simulink library "lib_all"  (Simulink 7.6)
settings: Solver=ode45  SolverMode=SingleTasking  StartTime=0.0  StopTime=10.0  RelTol=1e-3
values from neighbouring files (symbols this model uses):
  map_lib_03 = [6.8679 7.0159 7.4571 8.1849 9.1892 10.456 11.97 13.71 15.655 17.781 20.063 22.474 ... (803 elements, 73x11)]    [scr_simdata.mat]    (also in scr_simdata_all.mat)
  map_lib_06 = [2.5784 2.6727 2.9588 3.4453 4.1444 5.068 6.226 7.6242 9.2634 11.139 13.24 15.552 ... (803 elements, 73x11)]    [scr_simdata.mat]    (also in scr_simdata_all.mat)
  map_lib_09 = [0.0025784 0.0026727 0.0029588 0.0034453 0.0041444 0.005068 0.006226 0.0076242 0.0092634 0.011139 0.01324 0.015552 ... (803 elements, 73x11)]    [scr_simdata.mat]    (also in scr_simdata_all.mat)
  map_lib_10 = [2.74 2.75 2.76 2.77 2.78 2.79 2.8 2.81 2.82 2.83 2.84 2.85 ... (81 elements, 1x81)]    [scr_simdata.mat]    (also in scr_simdata_all.mat)
  map_lib_11 = [1.1983 1.2027 1.207 1.2113 1.2156 1.2198 1.2241 1.2283 1.2326 1.2368 1.241 1.2451 ... (81 elements, 1x81)]    [scr_simdata.mat]    (also in scr_simdata_all.mat)
  map_lib_12 = [2.74 2.75 2.76 2.77 2.78 2.79 2.8 2.81 2.82 2.83 2.84 2.85 ... (81 elements, 1x81)]    [scr_simdata.mat]    (also in scr_simdata_all.mat)
  map_lib_13 = [1.74 1.75 1.76 1.77 1.78 1.79 1.8 1.81 1.82 1.83 1.84 1.85 ... (81 elements, 1x81)]    [scr_simdata.mat]    (also in scr_simdata_all.mat)
  map_lib_16 = [0.00048828125 1.6159 1.8962 2.0821 2.2251 2.3426 2.4433 2.5318 2.611 2.683 2.749 2.8101 ... (1419 elements, 129x11)]    [scr_simdata.mat]    (also in scr_simdata_all.mat)
  map_mr2_01 = [0 800 1600 2400 3200 4000]    [scr_simdata.mat]    (also in scr_simdata_all.mat)
  map_mr2_02 = [1.32 1.32 1.33 1.34 1.36 1.38]    [scr_simdata.mat]    (also in scr_simdata_all.mat)
  map_mr2_03 = [0 800 1600 2400 3200 4000]    [scr_simdata.mat]    (also in scr_simdata_all.mat)
  map_mr2_04 = [1.32 1.32 1.33 1.34 1.36 1.38]    [scr_simdata.mat]    (also in scr_simdata_all.mat)
  map_mr2_05 = [0 800 1600 2400 3200 4000]    [scr_simdata.mat]    (also in scr_simdata_all.mat)
  map_mr2_06 = [1.32 1.32 1.33 1.34 1.36 1.38]    [scr_simdata.mat]    (also in scr_simdata_all.mat)
  Parameter1 = 6    [scr_simdata.mat]    (also in scr_simdata_all.mat)
  Parameter101 = 30    [scr_simdata.mat]    (also in scr_simdata_all.mat)
  Parameter102 = 12    [scr_simdata.mat]    (also in scr_simdata_all.mat)
  Parameter103 = -240    [scr_simdata.mat]    (also in scr_simdata_all.mat)
  Parameter108 = 48    [scr_simdata.mat]    (also in scr_simdata_all.mat)
  Parameter120 = 16    [scr_simdata.mat]    (also in scr_simdata_all.mat)
  Parameter121 = 87    [scr_simdata.mat]    (also in scr_simdata_all.mat)
  Parameter123 = 78    [scr_simdata.mat]    (also in scr_simdata_all.mat)
  Parameter124 = 60    [scr_simdata.mat]    (also in scr_simdata_all.mat)
  Parameter125 = -480    [scr_simdata.mat]    (also in scr_simdata_all.mat)
  Parameter130 = 96    [scr_simdata.mat]    (also in scr_simdata_all.mat)
  Parameter131 = 3    [scr_simdata.mat]    (also in scr_simdata_all.mat)
  Parameter144 = 3    [scr_simdata.mat]    (also in scr_simdata_all.mat)
  Parameter18 = 128    [scr_simdata.mat]    (also in scr_simdata_all.mat)
  Parameter19 = 16    [scr_simdata.mat]    (also in scr_simdata_all.mat)
  Parameter2 = 12    [scr_simdata.mat]    (also in scr_simdata_all.mat)
  Parameter20 = 7    [scr_simdata.mat]    (also in scr_simdata_all.mat)
  Parameter21 = 4    [scr_simdata.mat]    (also in scr_simdata_all.mat)
  Parameter3 = 18    [scr_simdata.mat]    (also in scr_simdata_all.mat)
  Parameter32 = 1    [scr_simdata.mat]    (also in scr_simdata_all.mat)
  Parameter34 = 24    [scr_simdata.mat]    (also in scr_simdata_all.mat)
  Parameter4 = 138    [scr_simdata.mat]    (also in scr_simdata_all.mat)
  Parameter42 = 48    [scr_simdata.mat]    (also in scr_simdata_all.mat)
  Parameter5 = 135    [scr_simdata.mat]    (also in scr_simdata_all.mat)
  Parameter55 = 0    [scr_simdata.mat]    (also in scr_simdata_all.mat)
  Parameter56 = 0    [scr_simdata.mat]    (also in scr_simdata_all.mat)
  Parameter57 = 1    [scr_simdata.mat]    (also in scr_simdata_all.mat)
  Parameter58 = 1    [scr_simdata.mat]    (also in scr_simdata_all.mat)
  Parameter59 = 2    [scr_simdata.mat]    (also in scr_simdata_all.mat)
  Parameter6 = 132    [scr_simdata.mat]    (also in scr_simdata_all.mat)
  Parameter60 = 2    [scr_simdata.mat]    (also in scr_simdata_all.mat)
  Parameter63 = 144    [scr_simdata.mat]    (also in scr_simdata_all.mat)
  Parameter7 = 126    [scr_simdata.mat]    (also in scr_simdata_all.mat)
  Parameter76 = 64    [scr_simdata.mat]    (also in scr_simdata_all.mat)
  Parameter77 = 135    [scr_simdata.mat]    (also in scr_simdata_all.mat)
  Parameter79 = 126    [scr_simdata.mat]    (also in scr_simdata_all.mat)
  Parameter80 = 108    [scr_simdata.mat]    (also in scr_simdata_all.mat)
  Parameter81 = 0    [scr_simdata.mat]    (also in scr_simdata_all.mat)
  Parameter86 = 0    [scr_simdata.mat]    (also in scr_simdata_all.mat)
  Parameter98 = 112    [scr_simdata.mat]    (also in scr_simdata_all.mat)
  Parameter99 = 39    [scr_simdata.mat]    (also in scr_simdata_all.mat)
undefined symbols (not defined in the file or in its folder): cylvolkp_hih_map2h, cylvolkp_map2h, pk1k_map2h
libraries: simulink x2
(part 31 of 36)

== system lib_all/SubSystem1196/SubSystem1273/SubSystem1275/SubSystem1284/SubSystem1308/SubSystem1314/SubSystem1316 ==
  note: ***編集禁止***
  element : Inport
  Inside_DSR : DataStoreRead  DataStoreName=DSM6
  Selector : Selector  IndexOptions=Index vector (port)  Indices=[1 3]  OutputSizes=1
  Output : Outport
  wires:
  Inside_DSR/1 -> Selector/1
  element/1 -> Selector/2
  Selector/1 -> Output/1

== system lib_all/SubSystem1196/SubSystem1273/SubSystem1275/SubSystem1284/SubSystem1308/SubSystem1314/SubSystem1317 ==
  note: ***編集禁止***
  element : Inport
  Inside_DSR : DataStoreRead  DataStoreName=DSM19
  Selector : Selector  IndexOptions=Index vector (port)  Indices=[1 3]  OutputSizes=1
  Output : Outport
  wires:
  Selector/1 -> Output/1
  element/1 -> Selector/2
  Inside_DSR/1 -> Selector/1

== system lib_all/SubSystem1196/SubSystem1273/SubSystem1275/SubSystem1284/SubSystem1318/SubSystem1320/SubSystem1321 ==
  note: ***編集禁止***
  element : Inport
  Inside_DSR : DataStoreRead  DataStoreName=DSM7
  Selector : Selector  IndexOptions=Index vector (port)  Indices=[1 3]  OutputSizes=1
  Output : Outport
  wires:
  Selector/1 -> Output/1
  element/1 -> Selector/2
  Inside_DSR/1 -> Selector/1

== system lib_all/SubSystem1196/SubSystem1273/SubSystem1275/SubSystem1284/SubSystem1318/SubSystem1320/SubSystem1322 ==
  note: ***編集禁止***
  element : Inport
  Inside_DSR : DataStoreRead  DataStoreName=DSM6
  Selector : Selector  IndexOptions=Index vector (port)  Indices=[1 3]  OutputSizes=1
  Output : Outport
  wires:
  Inside_DSR/1 -> Selector/1
  element/1 -> Selector/2
  Selector/1 -> Output/1

== system lib_all/SubSystem1196/SubSystem1273/SubSystem1275/SubSystem1284/SubSystem1318/SubSystem1320/SubSystem1323 ==
  note: ***編集禁止***
  element : Inport
  Inside_DSR : DataStoreRead  DataStoreName=DSM19
  Selector : Selector  IndexOptions=Index vector (port)  Indices=[1 3]  OutputSizes=1
  Output : Outport
  wires:
  Selector/1 -> Output/1
  element/1 -> Selector/2
  Inside_DSR/1 -> Selector/1

== system lib_all/SubSystem1196/SubSystem1273/SubSystem1275/SubSystem1284/SubSystem1318/SubSystem1324/SubSystem1325 ==
  note: ***編集禁止***
  element : Inport
  Inside_DSR : DataStoreRead  DataStoreName=DSM7
  Selector : Selector  IndexOptions=Index vector (port)  Indices=[1 3]  OutputSizes=1
  Output : Outport
  wires:
  Selector/1 -> Output/1
  element/1 -> Selector/2
  Inside_DSR/1 -> Selector/1

== system lib_all/SubSystem1196/SubSystem1273/SubSystem1275/SubSystem1284/SubSystem1318/SubSystem1324/SubSystem1326 ==
  note: ***編集禁止***
  element : Inport
  Inside_DSR : DataStoreRead  DataStoreName=DSM6
  Selector : Selector  IndexOptions=Index vector (port)  Indices=[1 3]  OutputSizes=1
  Output : Outport
  wires:
  Inside_DSR/1 -> Selector/1
  element/1 -> Selector/2
  Selector/1 -> Output/1

== system lib_all/SubSystem1196/SubSystem1273/SubSystem1275/SubSystem1284/SubSystem1318/SubSystem1324/SubSystem1327 ==
  note: ***編集禁止***
  element : Inport
  Inside_DSR : DataStoreRead  DataStoreName=DSM19
  Selector : Selector  IndexOptions=Index vector (port)  Indices=[1 3]  OutputSizes=1
  Output : Outport
  wires:
  Selector/1 -> Output/1
  element/1 -> Selector/2
  Inside_DSR/1 -> Selector/1

== system lib_all/SubSystem1196/SubSystem1273/SubSystem1328/SubSystem1330/SubSystem1331/SubSystem1334/SubSystem1335 ==
  note: ***編集禁止***
  In1 : Inport
  In2 : Inport  Port=2
  LO1 : Logic
  RO1 : RelationalOperator  Operator=<  InputSameDT=on
  RO2 : RelationalOperator  Operator=~=  InputSameDT=on
  single1 : DataTypeConversion  OutDataTypeStr=single  SaturateOnIntegerOverflow=on  RndMeth=Zero
  single2 : DataTypeConversion  OutDataTypeStr=single  SaturateOnIntegerOverflow=on  RndMeth=Zero
  Out1 : Outport
  wires:
  In2/1 -> single2/1
  In1/1 -> single1/1
  single1/1 -> RO1/1, RO2/1
  single2/1 -> RO1/2, RO2/2
  RO1/1 -> LO1/1
  RO2/1 -> LO1/2
  LO1/1 -> Out1/1

== system lib_all/SubSystem176/SubSystem178/SubSystem182/SubSystem183/SubSystem186/SubSystem187/Product ==
  note: ***編集禁止***
  mult : Inport
  div : Inport  Port=2
  init : Inport  Port=3
  Constant1 : Constant  OutDataTypeStr=Inherit: Inherit via back propagation
  Constant2 : Constant  Value=0  OutDataTypeStr=Inherit: Inherit via back propagation
  Product : Product  Inputs=*/  SaturateOnIntegerOverflow=on  RndMeth=Zero  InputSameDT=on  OutDataTypeStr=Inherit: Same as first input
  Relational Operator : RelationalOperator  Operator=~=  InputSameDT=on
  Switch : Switch  Threshold=0.5  SaturateOnIntegerOverflow=on  InputSameDT=on
  Switch1 : Switch  Threshold=0.5  SaturateOnIntegerOverflow=on  InputSameDT=on
  Out : Outport
  wires:
  Constant1/1 -> Switch1/3
  Constant2/1 -> Relational Operator/2
  Switch1/1 -> Product/2
  div/1 -> Relational Operator/1, Switch1/1
  Switch/1 -> Out/1
  init/1 -> Switch/3
  Relational Operator/1 -> Switch1/2, Switch/2
  Product/1 -> Switch/1
  mult/1 -> Product/1

== system lib_all/SubSystem176/SubSystem178/SubSystem182/SubSystem183/SubSystem186/SubSystem189/SubSystem190 ==
  note: ***編集禁止***
  In1 : Inport
  In2 : Inport  Port=2
  RO1 : RelationalOperator  Operator=>  InputSameDT=on
  single1 : DataTypeConversion  OutDataTypeStr=single  SaturateOnIntegerOverflow=on  RndMeth=Zero
  single2 : DataTypeConversion  OutDataTypeStr=single  SaturateOnIntegerOverflow=on  RndMeth=Zero
  Out1 : Outport
  wires:
  RO1/1 -> Out1/1
  In2/1 -> single2/1
  In1/1 -> single1/1
  single1/1 -> RO1/1
  single2/1 -> RO1/2

== system lib_all/SubSystem176/SubSystem178/SubSystem182/SubSystem183/SubSystem186/SubSystem189/SubSystem191 ==
  note: ***編集禁止***
  In1 : Inport
  In2 : Inport  Port=2
  LO1 : Logic
  RO1 : RelationalOperator  Operator=<  InputSameDT=on
  RO2 : RelationalOperator  Operator=~=  InputSameDT=on
  single1 : DataTypeConversion  OutDataTypeStr=single  SaturateOnIntegerOverflow=on  RndMeth=Zero
  single2 : DataTypeConversion  OutDataTypeStr=single  SaturateOnIntegerOverflow=on  RndMeth=Zero
  Out1 : Outport
  wires:
  In2/1 -> single2/1
  In1/1 -> single1/1
  single1/1 -> RO1/1, RO2/1
  single2/1 -> RO1/2, RO2/2
  RO1/1 -> LO1/1
  RO2/1 -> LO1/2
  LO1/1 -> Out1/1

== system lib_all/SubSystem176/SubSystem178/SubSystem182/SubSystem183/SubSystem192/SubSystem197/SubSystem198 ==
  Block478 : Inport
  Block479 : Inport  Port=2
  Block480 : MultiPortSwitch  zeroidx=off  RndMeth=Zero  SaturateOnIntegerOverflow=on  InputSameDT=on
  SubSystem199 : Subsystem  TreatAsAtomicUnit=on  mask(***ATC.DSRVEC***){bd_version=1.01.A, r0=DSM7, r1=-1, r2=, r3=, r4=, r5=, r6=, r7=, r8=, r9=, c0=, c1=, c2=, c3=, c4=, c5=, c6=, c7=, c8=, c9=}
      mask prompts: bd_version "Version"
      mask help: これはAutoCからのブロックです．編集禁止．
      settings: OpenFcn=warndlg('変更禁止!');
      mask Help: web(ui_blockhelpmng(gcb));
      mask Display: disp('ATC.DSRVEC')
  SubSystem200 : Subsystem  TreatAsAtomicUnit=on  mask(***ATC.DSRVEC***){bd_version=1.01.A, r0=DSM6, r1=-1, r2=, r3=, r4=, r5=, r6=, r7=, r8=, r9=, c0=, c1=, c2=, c3=, c4=, c5=, c6=, c7=, c8=, c9=}
      mask prompts: bd_version "Version"
      mask help: これはAutoCからのブロックです．編集禁止．
      settings: OpenFcn=warndlg('変更禁止!');
      mask Help: web(ui_blockhelpmng(gcb));
      mask Display: disp('ATC.DSRVEC')
  SubSystem201 : Subsystem  TreatAsAtomicUnit=on  mask(***ATC.DSRVEC***){bd_version=1.01.A, r0=DSM19, r1=-1, r2=, r3=, r4=, r5=, r6=, r7=, r8=, r9=, c0=, c1=, c2=, c3=, c4=, c5=, c6=, c7=, c8=, c9=}
      mask prompts: bd_version "Version"
      mask help: これはAutoCからのブロックです．編集禁止．
      settings: OpenFcn=warndlg('変更禁止!');
      mask Help: web(ui_blockhelpmng(gcb));
      mask Display: disp('ATC.DSRVEC')
  Block481 : Outport
  wires:
  Block479/1 -> SubSystem201/1(element), SubSystem200/1(element), SubSystem199/1(element)
  SubSystem201/1(Output) -> Block480/4
  SubSystem200/1(Output) -> Block480/3
  SubSystem199/1(Output) -> Block480/2
  Block480/1 -> Block481/1
  Block478/1 -> Block480/1

== system lib_all/SubSystem176/SubSystem178/SubSystem182/SubSystem183/SubSystem192/SubSystem197/SubSystem202 ==
  Block482 : Inport
  Block483 : Inport  Port=2
  Block484 : MultiPortSwitch  zeroidx=off  RndMeth=Zero  SaturateOnIntegerOverflow=on  InputSameDT=on
  SubSystem203 : Subsystem  TreatAsAtomicUnit=on  mask(***ATC.DSRVEC***){bd_version=1.01.A, r0=DSM7, r1=-1, r2=, r3=, r4=, r5=, r6=, r7=, r8=, r9=, c0=, c1=, c2=, c3=, c4=, c5=, c6=, c7=, c8=, c9=}
      mask prompts: bd_version "Version"
      mask help: これはAutoCからのブロックです．編集禁止．
      settings: OpenFcn=warndlg('変更禁止!');
      mask Help: web(ui_blockhelpmng(gcb));
      mask Display: disp('ATC.DSRVEC')
  SubSystem204 : Subsystem  TreatAsAtomicUnit=on  mask(***ATC.DSRVEC***){bd_version=1.01.A, r0=DSM6, r1=-1, r2=, r3=, r4=, r5=, r6=, r7=, r8=, r9=, c0=, c1=, c2=, c3=, c4=, c5=, c6=, c7=, c8=, c9=}
      mask prompts: bd_version "Version"
      mask help: これはAutoCからのブロックです．編集禁止．
      settings: OpenFcn=warndlg('変更禁止!');
      mask Help: web(ui_blockhelpmng(gcb));
      mask Display: disp('ATC.DSRVEC')
  SubSystem205 : Subsystem  TreatAsAtomicUnit=on  mask(***ATC.DSRVEC***){bd_version=1.01.A, r0=DSM19, r1=-1, r2=, r3=, r4=, r5=, r6=, r7=, r8=, r9=, c0=, c1=, c2=, c3=, c4=, c5=, c6=, c7=, c8=, c9=}
      mask prompts: bd_version "Version"
      mask help: これはAutoCからのブロックです．編集禁止．
      settings: OpenFcn=warndlg('変更禁止!');
      mask Help: web(ui_blockhelpmng(gcb));
      mask Display: disp('ATC.DSRVEC')
  Block485 : Outport
  wires:
  Block483/1 -> SubSystem205/1(element), SubSystem204/1(element), SubSystem203/1(element)
  SubSystem205/1(Output) -> Block484/4
  SubSystem204/1(Output) -> Block484/3
  SubSystem203/1(Output) -> Block484/2
  Block484/1 -> Block485/1
  Block482/1 -> Block484/1

== system lib_all/SubSystem176/SubSystem178/SubSystem182/SubSystem183/SubSystem192/SubSystem206/SubSystem207 ==
  note: ***編集禁止***
  element : Inport
  Inside_DSR : DataStoreRead  DataStoreName=DSM1
  Selector : Selector  IndexOptions=Index vector (port)  Indices=[1 3]  OutputSizes=1
  Output : Outport
  wires:
  Selector/1 -> Output/1
  element/1 -> Selector/2
  Inside_DSR/1 -> Selector/1

== system lib_all/SubSystem176/SubSystem178/SubSystem182/SubSystem183/SubSystem192/SubSystem206/SubSystem208 ==
  Block493 : Inport
  Block494 : Inport  Port=2
  Block495 : MultiPortSwitch  zeroidx=off  RndMeth=Zero  SaturateOnIntegerOverflow=on  InputSameDT=on
  SubSystem209 : Subsystem  TreatAsAtomicUnit=on  mask(***ATC.DSRVEC***){bd_version=1.01.A, r0=DSM7, r1=-1, r2=, r3=, r4=, r5=, r6=, r7=, r8=, r9=, c0=, c1=, c2=, c3=, c4=, c5=, c6=, c7=, c8=, c9=}
      mask prompts: bd_version "Version"
      mask help: これはAutoCからのブロックです．編集禁止．
      settings: OpenFcn=warndlg('変更禁止!');
      mask Help: web(ui_blockhelpmng(gcb));
      mask Display: disp('ATC.DSRVEC')
  SubSystem210 : Subsystem  TreatAsAtomicUnit=on  mask(***ATC.DSRVEC***){bd_version=1.01.A, r0=DSM6, r1=-1, r2=, r3=, r4=, r5=, r6=, r7=, r8=, r9=, c0=, c1=, c2=, c3=, c4=, c5=, c6=, c7=, c8=, c9=}
      mask prompts: bd_version "Version"
      mask help: これはAutoCからのブロックです．編集禁止．
      settings: OpenFcn=warndlg('変更禁止!');
      mask Help: web(ui_blockhelpmng(gcb));
      mask Display: disp('ATC.DSRVEC')
  SubSystem211 : Subsystem  TreatAsAtomicUnit=on  mask(***ATC.DSRVEC***){bd_version=1.01.A, r0=DSM19, r1=-1, r2=, r3=, r4=, r5=, r6=, r7=, r8=, r9=, c0=, c1=, c2=, c3=, c4=, c5=, c6=, c7=, c8=, c9=}
      mask prompts: bd_version "Version"
      mask help: これはAutoCからのブロックです．編集禁止．
      settings: OpenFcn=warndlg('変更禁止!');
      mask Help: web(ui_blockhelpmng(gcb));
      mask Display: disp('ATC.DSRVEC')
  Block496 : Outport
  wires:
  Block494/1 -> SubSystem211/1(element), SubSystem210/1(element), SubSystem209/1(element)
  SubSystem211/1(Output) -> Block495/4
  SubSystem210/1(Output) -> Block495/3
  SubSystem209/1(Output) -> Block495/2
  Block495/1 -> Block496/1
  Block493/1 -> Block495/1

== system lib_all/SubSystem176/SubSystem178/SubSystem182/SubSystem183/SubSystem192/SubSystem206/SubSystem212 ==
  Block497 : Inport
  Block498 : Inport  Port=2
  Block499 : MultiPortSwitch  zeroidx=off  RndMeth=Zero  SaturateOnIntegerOverflow=on  InputSameDT=on
  SubSystem213 : Subsystem  TreatAsAtomicUnit=on  mask(***ATC.DSRVEC***){bd_version=1.01.A, r0=DSM7, r1=-1, r2=, r3=, r4=, r5=, r6=, r7=, r8=, r9=, c0=, c1=, c2=, c3=, c4=, c5=, c6=, c7=, c8=, c9=}
      mask prompts: bd_version "Version"
      mask help: これはAutoCからのブロックです．編集禁止．
      settings: OpenFcn=warndlg('変更禁止!');
      mask Help: web(ui_blockhelpmng(gcb));
      mask Display: disp('ATC.DSRVEC')
  SubSystem214 : Subsystem  TreatAsAtomicUnit=on  mask(***ATC.DSRVEC***){bd_version=1.01.A, r0=DSM6, r1=-1, r2=, r3=, r4=, r5=, r6=, r7=, r8=, r9=, c0=, c1=, c2=, c3=, c4=, c5=, c6=, c7=, c8=, c9=}
      mask prompts: bd_version "Version"
      mask help: これはAutoCからのブロックです．編集禁止．
      settings: OpenFcn=warndlg('変更禁止!');
      mask Help: web(ui_blockhelpmng(gcb));
      mask Display: disp('ATC.DSRVEC')
  SubSystem215 : Subsystem  TreatAsAtomicUnit=on  mask(***ATC.DSRVEC***){bd_version=1.01.A, r0=DSM19, r1=-1, r2=, r3=, r4=, r5=, r6=, r7=, r8=, r9=, c0=, c1=, c2=, c3=, c4=, c5=, c6=, c7=, c8=, c9=}
      mask prompts: bd_version "Version"
      mask help: これはAutoCからのブロックです．編集禁止．
      settings: OpenFcn=warndlg('変更禁止!');
      mask Help: web(ui_blockhelpmng(gcb));
      mask Display: disp('ATC.DSRVEC')
  Block500 : Outport
  wires:
  Block498/1 -> SubSystem215/1(element), SubSystem214/1(element), SubSystem213/1(element)
  SubSystem215/1(Output) -> Block499/4
  SubSystem214/1(Output) -> Block499/3
  SubSystem213/1(Output) -> Block499/2
  Block499/1 -> Block500/1
  Block497/1 -> Block499/1

== system lib_all/SubSystem176/SubSystem178/SubSystem182/SubSystem183/SubSystem192/SubSystem216/SubSystem217 ==
  note: ***編集禁止***
  element : Inport
  Inside_DSR : DataStoreRead  DataStoreName=DSM5
  Selector : Selector  IndexOptions=Index vector (port)  Indices=[1 3]  OutputSizes=1
  Output : Outport
  wires:
  Selector/1 -> Output/1
  element/1 -> Selector/2
  Inside_DSR/1 -> Selector/1

== system lib_all/SubSystem176/SubSystem178/SubSystem182/SubSystem183/SubSystem192/SubSystem216/SubSystem218 ==
  Block508 : Inport
  Block509 : Inport  Port=2
  Block510 : MultiPortSwitch  zeroidx=off  RndMeth=Zero  SaturateOnIntegerOverflow=on  InputSameDT=on
  SubSystem219 : Subsystem  TreatAsAtomicUnit=on  mask(***ATC.DSRVEC***){bd_version=1.01.A, r0=DSM7, r1=-1, r2=, r3=, r4=, r5=, r6=, r7=, r8=, r9=, c0=, c1=, c2=, c3=, c4=, c5=, c6=, c7=, c8=, c9=}
      mask prompts: bd_version "Version"
      mask help: これはAutoCからのブロックです．編集禁止．
      settings: OpenFcn=warndlg('変更禁止!');
      mask Help: web(ui_blockhelpmng(gcb));
      mask Display: disp('ATC.DSRVEC')
  SubSystem220 : Subsystem  TreatAsAtomicUnit=on  mask(***ATC.DSRVEC***){bd_version=1.01.A, r0=DSM6, r1=-1, r2=, r3=, r4=, r5=, r6=, r7=, r8=, r9=, c0=, c1=, c2=, c3=, c4=, c5=, c6=, c7=, c8=, c9=}
      mask prompts: bd_version "Version"
      mask help: これはAutoCからのブロックです．編集禁止．
      settings: OpenFcn=warndlg('変更禁止!');
      mask Help: web(ui_blockhelpmng(gcb));
      mask Display: disp('ATC.DSRVEC')
  SubSystem221 : Subsystem  TreatAsAtomicUnit=on  mask(***ATC.DSRVEC***){bd_version=1.01.A, r0=DSM19, r1=-1, r2=, r3=, r4=, r5=, r6=, r7=, r8=, r9=, c0=, c1=, c2=, c3=, c4=, c5=, c6=, c7=, c8=, c9=}
      mask prompts: bd_version "Version"
      mask help: これはAutoCからのブロックです．編集禁止．
      settings: OpenFcn=warndlg('変更禁止!');
      mask Help: web(ui_blockhelpmng(gcb));
      mask Display: disp('ATC.DSRVEC')
  Block511 : Outport
  wires:
  Block509/1 -> SubSystem221/1(element), SubSystem220/1(element), SubSystem219/1(element)
  SubSystem221/1(Output) -> Block510/4
  SubSystem220/1(Output) -> Block510/3
  SubSystem219/1(Output) -> Block510/2
  Block510/1 -> Block511/1
  Block508/1 -> Block510/1

== system lib_all/SubSystem176/SubSystem178/SubSystem182/SubSystem183/SubSystem192/SubSystem216/SubSystem222 ==
  Block512 : Inport
  Block513 : Inport  Port=2
  Block514 : MultiPortSwitch  zeroidx=off  RndMeth=Zero  SaturateOnIntegerOverflow=on  InputSameDT=on
  SubSystem223 : Subsystem  TreatAsAtomicUnit=on  mask(***ATC.DSRVEC***){bd_version=1.01.A, r0=DSM7, r1=-1, r2=, r3=, r4=, r5=, r6=, r7=, r8=, r9=, c0=, c1=, c2=, c3=, c4=, c5=, c6=, c7=, c8=, c9=}
      mask prompts: bd_version "Version"
      mask help: これはAutoCからのブロックです．編集禁止．
      settings: OpenFcn=warndlg('変更禁止!');
      mask Help: web(ui_blockhelpmng(gcb));
      mask Display: disp('ATC.DSRVEC')
  SubSystem224 : Subsystem  TreatAsAtomicUnit=on  mask(***ATC.DSRVEC***){bd_version=1.01.A, r0=DSM6, r1=-1, r2=, r3=, r4=, r5=, r6=, r7=, r8=, r9=, c0=, c1=, c2=, c3=, c4=, c5=, c6=, c7=, c8=, c9=}
      mask prompts: bd_version "Version"
      mask help: これはAutoCからのブロックです．編集禁止．
      settings: OpenFcn=warndlg('変更禁止!');
      mask Help: web(ui_blockhelpmng(gcb));
      mask Display: disp('ATC.DSRVEC')
  SubSystem225 : Subsystem  TreatAsAtomicUnit=on  mask(***ATC.DSRVEC***){bd_version=1.01.A, r0=DSM19, r1=-1, r2=, r3=, r4=, r5=, r6=, r7=, r8=, r9=, c0=, c1=, c2=, c3=, c4=, c5=, c6=, c7=, c8=, c9=}
      mask prompts: bd_version "Version"
      mask help: これはAutoCからのブロックです．編集禁止．
      settings: OpenFcn=warndlg('変更禁止!');
      mask Help: web(ui_blockhelpmng(gcb));
      mask Display: disp('ATC.DSRVEC')
  Block515 : Outport
  wires:
  Block513/1 -> SubSystem225/1(element), SubSystem224/1(element), SubSystem223/1(element)
  SubSystem225/1(Output) -> Block514/4
  SubSystem224/1(Output) -> Block514/3
  SubSystem223/1(Output) -> Block514/2
  Block514/1 -> Block515/1
  Block512/1 -> Block514/1

== system lib_all/SubSystem176/SubSystem178/SubSystem182/SubSystem183/SubSystem192/SubSystem226/SubSystem227 ==
  note: ***編集禁止***
  element : Inport
  Inside_DSR : DataStoreRead  DataStoreName=DSM3
  Selector : Selector  IndexOptions=Index vector (port)  Indices=[1 3]  OutputSizes=1
  Output : Outport
  wires:
  Selector/1 -> Output/1
  element/1 -> Selector/2
  Inside_DSR/1 -> Selector/1

== system lib_all/SubSystem176/SubSystem178/SubSystem182/SubSystem183/SubSystem192/SubSystem226/SubSystem228 ==
  Block523 : Inport
  Block524 : Inport  Port=2
  Block525 : MultiPortSwitch  zeroidx=off  RndMeth=Zero  SaturateOnIntegerOverflow=on  InputSameDT=on
  SubSystem229 : Subsystem  TreatAsAtomicUnit=on  mask(***ATC.DSRVEC***){bd_version=1.01.A, r0=DSM7, r1=-1, r2=, r3=, r4=, r5=, r6=, r7=, r8=, r9=, c0=, c1=, c2=, c3=, c4=, c5=, c6=, c7=, c8=, c9=}
      mask prompts: bd_version "Version"
      mask help: これはAutoCからのブロックです．編集禁止．
      settings: OpenFcn=warndlg('変更禁止!');
      mask Help: web(ui_blockhelpmng(gcb));
      mask Display: disp('ATC.DSRVEC')
  SubSystem230 : Subsystem  TreatAsAtomicUnit=on  mask(***ATC.DSRVEC***){bd_version=1.01.A, r0=DSM6, r1=-1, r2=, r3=, r4=, r5=, r6=, r7=, r8=, r9=, c0=, c1=, c2=, c3=, c4=, c5=, c6=, c7=, c8=, c9=}
      mask prompts: bd_version "Version"
      mask help: これはAutoCからのブロックです．編集禁止．
      settings: OpenFcn=warndlg('変更禁止!');
      mask Help: web(ui_blockhelpmng(gcb));
      mask Display: disp('ATC.DSRVEC')
  SubSystem231 : Subsystem  TreatAsAtomicUnit=on  mask(***ATC.DSRVEC***){bd_version=1.01.A, r0=DSM19, r1=-1, r2=, r3=, r4=, r5=, r6=, r7=, r8=, r9=, c0=, c1=, c2=, c3=, c4=, c5=, c6=, c7=, c8=, c9=}
      mask prompts: bd_version "Version"
      mask help: これはAutoCからのブロックです．編集禁止．
      settings: OpenFcn=warndlg('変更禁止!');
      mask Help: web(ui_blockhelpmng(gcb));
      mask Display: disp('ATC.DSRVEC')
  Block526 : Outport
  wires:
  Block524/1 -> SubSystem231/1(element), SubSystem230/1(element), SubSystem229/1(element)
  SubSystem231/1(Output) -> Block525/4
  SubSystem230/1(Output) -> Block525/3
  SubSystem229/1(Output) -> Block525/2
  Block525/1 -> Block526/1
  Block523/1 -> Block525/1

== system lib_all/SubSystem176/SubSystem178/SubSystem182/SubSystem183/SubSystem192/SubSystem226/SubSystem232 ==
  Block527 : Inport
  Block528 : Inport  Port=2
  Block529 : MultiPortSwitch  zeroidx=off  RndMeth=Zero  SaturateOnIntegerOverflow=on  InputSameDT=on
  SubSystem233 : Subsystem  TreatAsAtomicUnit=on  mask(***ATC.DSRVEC***){bd_version=1.01.A, r0=DSM7, r1=-1, r2=, r3=, r4=, r5=, r6=, r7=, r8=, r9=, c0=, c1=, c2=, c3=, c4=, c5=, c6=, c7=, c8=, c9=}
      mask prompts: bd_version "Version"
      mask help: これはAutoCからのブロックです．編集禁止．
      settings: OpenFcn=warndlg('変更禁止!');
      mask Help: web(ui_blockhelpmng(gcb));
      mask Display: disp('ATC.DSRVEC')
  SubSystem234 : Subsystem  TreatAsAtomicUnit=on  mask(***ATC.DSRVEC***){bd_version=1.01.A, r0=DSM6, r1=-1, r2=, r3=, r4=, r5=, r6=, r7=, r8=, r9=, c0=, c1=, c2=, c3=, c4=, c5=, c6=, c7=, c8=, c9=}
      mask prompts: bd_version "Version"
      mask help: これはAutoCからのブロックです．編集禁止．
      settings: OpenFcn=warndlg('変更禁止!');
      mask Help: web(ui_blockhelpmng(gcb));
      mask Display: disp('ATC.DSRVEC')
  SubSystem235 : Subsystem  TreatAsAtomicUnit=on  mask(***ATC.DSRVEC***){bd_version=1.01.A, r0=DSM19, r1=-1, r2=, r3=, r4=, r5=, r6=, r7=, r8=, r9=, c0=, c1=, c2=, c3=, c4=, c5=, c6=, c7=, c8=, c9=}
      mask prompts: bd_version "Version"
      mask help: これはAutoCからのブロックです．編集禁止．
      settings: OpenFcn=warndlg('変更禁止!');
      mask Help: web(ui_blockhelpmng(gcb));
      mask Display: disp('ATC.DSRVEC')
  Block530 : Outport
  wires:
  Block528/1 -> SubSystem235/1(element), SubSystem234/1(element), SubSystem233/1(element)
  SubSystem235/1(Output) -> Block529/4
  SubSystem234/1(Output) -> Block529/3
  SubSystem233/1(Output) -> Block529/2
  Block529/1 -> Block530/1
  Block527/1 -> Block529/1

== system lib_all/SubSystem176/SubSystem178/SubSystem182/SubSystem236/SubSystem239/SubSystem240/Product ==
  note: ***編集禁止***
  mult : Inport
  div : Inport  Port=2
  init : Inport  Port=3
  Constant1 : Constant  OutDataTypeStr=Inherit: Inherit via back propagation
  Constant2 : Constant  Value=0  OutDataTypeStr=Inherit: Inherit via back propagation
  Product : Product  Inputs=*/  SaturateOnIntegerOverflow=on  RndMeth=Zero  InputSameDT=on  OutDataTypeStr=Inherit: Same as first input
  Relational Operator : RelationalOperator  Operator=~=  InputSameDT=on
  Switch : Switch  Threshold=0.5  SaturateOnIntegerOverflow=on  InputSameDT=on
  Switch1 : Switch  Threshold=0.5  SaturateOnIntegerOverflow=on  InputSameDT=on
  Out : Outport
  wires:
  Constant1/1 -> Switch1/3
  Constant2/1 -> Relational Operator/2
  Switch1/1 -> Product/2
  div/1 -> Relational Operator/1, Switch1/1
  Switch/1 -> Out/1
  init/1 -> Switch/3
  Relational Operator/1 -> Switch1/2, Switch/2
  Product/1 -> Switch/1
  mult/1 -> Product/1

== system lib_all/SubSystem176/SubSystem178/SubSystem182/SubSystem236/SubSystem239/SubSystem242/SubSystem243 ==
  note: ***編集禁止***
  In1 : Inport
  In2 : Inport  Port=2
  RO1 : RelationalOperator  Operator=>  InputSameDT=on
  single1 : DataTypeConversion  OutDataTypeStr=single  SaturateOnIntegerOverflow=on  RndMeth=Zero
  single2 : DataTypeConversion  OutDataTypeStr=single  SaturateOnIntegerOverflow=on  RndMeth=Zero
  Out1 : Outport
  wires:
  RO1/1 -> Out1/1
  In2/1 -> single2/1
  In1/1 -> single1/1
  single1/1 -> RO1/1
  single2/1 -> RO1/2

== system lib_all/SubSystem176/SubSystem178/SubSystem182/SubSystem236/SubSystem239/SubSystem242/SubSystem244 ==
  note: ***編集禁止***
  In1 : Inport
  In2 : Inport  Port=2
  LO1 : Logic
  RO1 : RelationalOperator  Operator=<  InputSameDT=on
  RO2 : RelationalOperator  Operator=~=  InputSameDT=on
  single1 : DataTypeConversion  OutDataTypeStr=single  SaturateOnIntegerOverflow=on  RndMeth=Zero
  single2 : DataTypeConversion  OutDataTypeStr=single  SaturateOnIntegerOverflow=on  RndMeth=Zero
  Out1 : Outport
  wires:
  In2/1 -> single2/1
  In1/1 -> single1/1
  single1/1 -> RO1/1, RO2/1
  single2/1 -> RO1/2, RO2/2
  RO1/1 -> LO1/1
  RO2/1 -> LO1/2
  LO1/1 -> Out1/1

== system lib_all/SubSystem176/SubSystem178/SubSystem182/SubSystem236/SubSystem245/SubSystem250/SubSystem251 ==
  Block600 : Inport
  Block601 : Inport  Port=2
  Block602 : MultiPortSwitch  zeroidx=off  RndMeth=Zero  SaturateOnIntegerOverflow=on  InputSameDT=on
  SubSystem252 : Subsystem  TreatAsAtomicUnit=on  mask(***ATC.DSRVEC***){bd_version=1.01.A, r0=DSM7, r1=-1, r2=, r3=, r4=, r5=, r6=, r7=, r8=, r9=, c0=, c1=, c2=, c3=, c4=, c5=, c6=, c7=, c8=, c9=}
      mask prompts: bd_version "Version"
      mask help: これはAutoCからのブロックです．編集禁止．
      settings: OpenFcn=warndlg('変更禁止!');
      mask Help: web(ui_blockhelpmng(gcb));
      mask Display: disp('ATC.DSRVEC')
  SubSystem253 : Subsystem  TreatAsAtomicUnit=on  mask(***ATC.DSRVEC***){bd_version=1.01.A, r0=DSM6, r1=-1, r2=, r3=, r4=, r5=, r6=, r7=, r8=, r9=, c0=, c1=, c2=, c3=, c4=, c5=, c6=, c7=, c8=, c9=}
      mask prompts: bd_version "Version"
      mask help: これはAutoCからのブロックです．編集禁止．
      settings: OpenFcn=warndlg('変更禁止!');
      mask Help: web(ui_blockhelpmng(gcb));
      mask Display: disp('ATC.DSRVEC')
  SubSystem254 : Subsystem  TreatAsAtomicUnit=on  mask(***ATC.DSRVEC***){bd_version=1.01.A, r0=DSM19, r1=-1, r2=, r3=, r4=, r5=, r6=, r7=, r8=, r9=, c0=, c1=, c2=, c3=, c4=, c5=, c6=, c7=, c8=, c9=}
      mask prompts: bd_version "Version"
      mask help: これはAutoCからのブロックです．編集禁止．
      settings: OpenFcn=warndlg('変更禁止!');
      mask Help: web(ui_blockhelpmng(gcb));
      mask Display: disp('ATC.DSRVEC')
  Block603 : Outport
  wires:
  Block601/1 -> SubSystem254/1(element), SubSystem253/1(element), SubSystem252/1(element)
  SubSystem254/1(Output) -> Block602/4
  SubSystem253/1(Output) -> Block602/3
  SubSystem252/1(Output) -> Block602/2
  Block602/1 -> Block603/1
  Block600/1 -> Block602/1

== system lib_all/SubSystem176/SubSystem178/SubSystem182/SubSystem236/SubSystem245/SubSystem250/SubSystem255 ==
  Block604 : Inport
  Block605 : Inport  Port=2
  Block606 : MultiPortSwitch  zeroidx=off  RndMeth=Zero  SaturateOnIntegerOverflow=on  InputSameDT=on
  SubSystem256 : Subsystem  TreatAsAtomicUnit=on  mask(***ATC.DSRVEC***){bd_version=1.01.A, r0=DSM7, r1=-1, r2=, r3=, r4=, r5=, r6=, r7=, r8=, r9=, c0=, c1=, c2=, c3=, c4=, c5=, c6=, c7=, c8=, c9=}
      mask prompts: bd_version "Version"
      mask help: これはAutoCからのブロックです．編集禁止．
      settings: OpenFcn=warndlg('変更禁止!');
      mask Help: web(ui_blockhelpmng(gcb));
      mask Display: disp('ATC.DSRVEC')
  SubSystem257 : Subsystem  TreatAsAtomicUnit=on  mask(***ATC.DSRVEC***){bd_version=1.01.A, r0=DSM6, r1=-1, r2=, r3=, r4=, r5=, r6=, r7=, r8=, r9=, c0=, c1=, c2=, c3=, c4=, c5=, c6=, c7=, c8=, c9=}
      mask prompts: bd_version "Version"
      mask help: これはAutoCからのブロックです．編集禁止．
      settings: OpenFcn=warndlg('変更禁止!');
      mask Help: web(ui_blockhelpmng(gcb));
      mask Display: disp('ATC.DSRVEC')
  SubSystem258 : Subsystem  TreatAsAtomicUnit=on  mask(***ATC.DSRVEC***){bd_version=1.01.A, r0=DSM19, r1=-1, r2=, r3=, r4=, r5=, r6=, r7=, r8=, r9=, c0=, c1=, c2=, c3=, c4=, c5=, c6=, c7=, c8=, c9=}
      mask prompts: bd_version "Version"
      mask help: これはAutoCからのブロックです．編集禁止．
      settings: OpenFcn=warndlg('変更禁止!');
      mask Help: web(ui_blockhelpmng(gcb));
      mask Display: disp('ATC.DSRVEC')
  Block607 : Outport
  wires:
  Block605/1 -> SubSystem258/1(element), SubSystem257/1(element), SubSystem256/1(element)
  SubSystem258/1(Output) -> Block606/4
  SubSystem257/1(Output) -> Block606/3
  SubSystem256/1(Output) -> Block606/2
  Block606/1 -> Block607/1
  Block604/1 -> Block606/1

== system lib_all/SubSystem176/SubSystem178/SubSystem182/SubSystem236/SubSystem245/SubSystem259/SubSystem260 ==
  note: ***編集禁止***
  element : Inport
  Inside_DSR : DataStoreRead  DataStoreName=DSM1
  Selector : Selector  IndexOptions=Index vector (port)  Indices=[1 3]  OutputSizes=1
  Output : Outport
  wires:
  Selector/1 -> Output/1
  element/1 -> Selector/2
  Inside_DSR/1 -> Selector/1

== system lib_all/SubSystem176/SubSystem178/SubSystem182/SubSystem236/SubSystem245/SubSystem259/SubSystem261 ==
  Block615 : Inport
  Block616 : Inport  Port=2
  Block617 : MultiPortSwitch  zeroidx=off  RndMeth=Zero  SaturateOnIntegerOverflow=on  InputSameDT=on
  SubSystem262 : Subsystem  TreatAsAtomicUnit=on  mask(***ATC.DSRVEC***){bd_version=1.01.A, r0=DSM7, r1=-1, r2=, r3=, r4=, r5=, r6=, r7=, r8=, r9=, c0=, c1=, c2=, c3=, c4=, c5=, c6=, c7=, c8=, c9=}
      mask prompts: bd_version "Version"
      mask help: これはAutoCからのブロックです．編集禁止．
      settings: OpenFcn=warndlg('変更禁止!');
      mask Help: web(ui_blockhelpmng(gcb));
      mask Display: disp('ATC.DSRVEC')
  SubSystem263 : Subsystem  TreatAsAtomicUnit=on  mask(***ATC.DSRVEC***){bd_version=1.01.A, r0=DSM6, r1=-1, r2=, r3=, r4=, r5=, r6=, r7=, r8=, r9=, c0=, c1=, c2=, c3=, c4=, c5=, c6=, c7=, c8=, c9=}
      mask prompts: bd_version "Version"
      mask help: これはAutoCからのブロックです．編集禁止．
      settings: OpenFcn=warndlg('変更禁止!');
      mask Help: web(ui_blockhelpmng(gcb));
      mask Display: disp('ATC.DSRVEC')
  SubSystem264 : Subsystem  TreatAsAtomicUnit=on  mask(***ATC.DSRVEC***){bd_version=1.01.A, r0=DSM19, r1=-1, r2=, r3=, r4=, r5=, r6=, r7=, r8=, r9=, c0=, c1=, c2=, c3=, c4=, c5=, c6=, c7=, c8=, c9=}
      mask prompts: bd_version "Version"
      mask help: これはAutoCからのブロックです．編集禁止．
      settings: OpenFcn=warndlg('変更禁止!');
      mask Help: web(ui_blockhelpmng(gcb));
      mask Display: disp('ATC.DSRVEC')
  Block618 : Outport
  wires:
  Block616/1 -> SubSystem264/1(element), SubSystem263/1(element), SubSystem262/1(element)
  SubSystem264/1(Output) -> Block617/4
  SubSystem263/1(Output) -> Block617/3
  SubSystem262/1(Output) -> Block617/2
  Block617/1 -> Block618/1
  Block615/1 -> Block617/1

== system lib_all/SubSystem176/SubSystem178/SubSystem182/SubSystem236/SubSystem245/SubSystem259/SubSystem265 ==
  Block619 : Inport
  Block620 : Inport  Port=2
  Block621 : MultiPortSwitch  zeroidx=off  RndMeth=Zero  SaturateOnIntegerOverflow=on  InputSameDT=on
  SubSystem266 : Subsystem  TreatAsAtomicUnit=on  mask(***ATC.DSRVEC***){bd_version=1.01.A, r0=DSM7, r1=-1, r2=, r3=, r4=, r5=, r6=, r7=, r8=, r9=, c0=, c1=, c2=, c3=, c4=, c5=, c6=, c7=, c8=, c9=}
      mask prompts: bd_version "Version"
      mask help: これはAutoCからのブロックです．編集禁止．
      settings: OpenFcn=warndlg('変更禁止!');
      mask Help: web(ui_blockhelpmng(gcb));
      mask Display: disp('ATC.DSRVEC')
  SubSystem267 : Subsystem  TreatAsAtomicUnit=on  mask(***ATC.DSRVEC***){bd_version=1.01.A, r0=DSM6, r1=-1, r2=, r3=, r4=, r5=, r6=, r7=, r8=, r9=, c0=, c1=, c2=, c3=, c4=, c5=, c6=, c7=, c8=, c9=}
      mask prompts: bd_version "Version"
      mask help: これはAutoCからのブロックです．編集禁止．
      settings: OpenFcn=warndlg('変更禁止!');
      mask Help: web(ui_blockhelpmng(gcb));
      mask Display: disp('ATC.DSRVEC')
  SubSystem268 : Subsystem  TreatAsAtomicUnit=on  mask(***ATC.DSRVEC***){bd_version=1.01.A, r0=DSM19, r1=-1, r2=, r3=, r4=, r5=, r6=, r7=, r8=, r9=, c0=, c1=, c2=, c3=, c4=, c5=, c6=, c7=, c8=, c9=}
      mask prompts: bd_version "Version"
      mask help: これはAutoCからのブロックです．編集禁止．
      settings: OpenFcn=warndlg('変更禁止!');
      mask Help: web(ui_blockhelpmng(gcb));
      mask Display: disp('ATC.DSRVEC')
  Block622 : Outport
  wires:
  Block620/1 -> SubSystem268/1(element), SubSystem267/1(element), SubSystem266/1(element)
  SubSystem268/1(Output) -> Block621/4
  SubSystem267/1(Output) -> Block621/3
  SubSystem266/1(Output) -> Block621/2
  Block621/1 -> Block622/1
  Block619/1 -> Block621/1

== system lib_all/SubSystem176/SubSystem178/SubSystem182/SubSystem236/SubSystem245/SubSystem269/SubSystem270 ==
  note: ***編集禁止***
  element : Inport
  Inside_DSR : DataStoreRead  DataStoreName=DSM5
  Selector : Selector  IndexOptions=Index vector (port)  Indices=[1 3]  OutputSizes=1
  Output : Outport
  wires:
  Selector/1 -> Output/1
  element/1 -> Selector/2
  Inside_DSR/1 -> Selector/1

== system lib_all/SubSystem176/SubSystem178/SubSystem182/SubSystem236/SubSystem245/SubSystem269/SubSystem271 ==
  Block630 : Inport
  Block631 : Inport  Port=2
  Block632 : MultiPortSwitch  zeroidx=off  RndMeth=Zero  SaturateOnIntegerOverflow=on  InputSameDT=on
  SubSystem272 : Subsystem  TreatAsAtomicUnit=on  mask(***ATC.DSRVEC***){bd_version=1.01.A, r0=DSM7, r1=-1, r2=, r3=, r4=, r5=, r6=, r7=, r8=, r9=, c0=, c1=, c2=, c3=, c4=, c5=, c6=, c7=, c8=, c9=}
      mask prompts: bd_version "Version"
      mask help: これはAutoCからのブロックです．編集禁止．
      settings: OpenFcn=warndlg('変更禁止!');
      mask Help: web(ui_blockhelpmng(gcb));
      mask Display: disp('ATC.DSRVEC')
  SubSystem273 : Subsystem  TreatAsAtomicUnit=on  mask(***ATC.DSRVEC***){bd_version=1.01.A, r0=DSM6, r1=-1, r2=, r3=, r4=, r5=, r6=, r7=, r8=, r9=, c0=, c1=, c2=, c3=, c4=, c5=, c6=, c7=, c8=, c9=}
      mask prompts: bd_version "Version"
      mask help: これはAutoCからのブロックです．編集禁止．
      settings: OpenFcn=warndlg('変更禁止!');
      mask Help: web(ui_blockhelpmng(gcb));
      mask Display: disp('ATC.DSRVEC')
  SubSystem274 : Subsystem  TreatAsAtomicUnit=on  mask(***ATC.DSRVEC***){bd_version=1.01.A, r0=DSM19, r1=-1, r2=, r3=, r4=, r5=, r6=, r7=, r8=, r9=, c0=, c1=, c2=, c3=, c4=, c5=, c6=, c7=, c8=, c9=}
      mask prompts: bd_version "Version"
      mask help: これはAutoCからのブロックです．編集禁止．
      settings: OpenFcn=warndlg('変更禁止!');
      mask Help: web(ui_blockhelpmng(gcb));
      mask Display: disp('ATC.DSRVEC')
  Block633 : Outport
  wires:
  Block631/1 -> SubSystem274/1(element), SubSystem273/1(element), SubSystem272/1(element)
  SubSystem274/1(Output) -> Block632/4
  SubSystem273/1(Output) -> Block632/3
  SubSystem272/1(Output) -> Block632/2
  Block632/1 -> Block633/1
  Block630/1 -> Block632/1

== system lib_all/SubSystem176/SubSystem178/SubSystem182/SubSystem236/SubSystem245/SubSystem269/SubSystem275 ==
  Block634 : Inport
  Block635 : Inport  Port=2
  Block636 : MultiPortSwitch  zeroidx=off  RndMeth=Zero  SaturateOnIntegerOverflow=on  InputSameDT=on
  SubSystem276 : Subsystem  TreatAsAtomicUnit=on  mask(***ATC.DSRVEC***){bd_version=1.01.A, r0=DSM7, r1=-1, r2=, r3=, r4=, r5=, r6=, r7=, r8=, r9=, c0=, c1=, c2=, c3=, c4=, c5=, c6=, c7=, c8=, c9=}
      mask prompts: bd_version "Version"
      mask help: これはAutoCからのブロックです．編集禁止．
      settings: OpenFcn=warndlg('変更禁止!');
      mask Help: web(ui_blockhelpmng(gcb));
      mask Display: disp('ATC.DSRVEC')
  SubSystem277 : Subsystem  TreatAsAtomicUnit=on  mask(***ATC.DSRVEC***){bd_version=1.01.A, r0=DSM6, r1=-1, r2=, r3=, r4=, r5=, r6=, r7=, r8=, r9=, c0=, c1=, c2=, c3=, c4=, c5=, c6=, c7=, c8=, c9=}
      mask prompts: bd_version "Version"
      mask help: これはAutoCからのブロックです．編集禁止．
      settings: OpenFcn=warndlg('変更禁止!');
      mask Help: web(ui_blockhelpmng(gcb));
      mask Display: disp('ATC.DSRVEC')
  SubSystem278 : Subsystem  TreatAsAtomicUnit=on  mask(***ATC.DSRVEC***){bd_version=1.01.A, r0=DSM19, r1=-1, r2=, r3=, r4=, r5=, r6=, r7=, r8=, r9=, c0=, c1=, c2=, c3=, c4=, c5=, c6=, c7=, c8=, c9=}
      mask prompts: bd_version "Version"
      mask help: これはAutoCからのブロックです．編集禁止．
      settings: OpenFcn=warndlg('変更禁止!');
      mask Help: web(ui_blockhelpmng(gcb));
      mask Display: disp('ATC.DSRVEC')
  Block637 : Outport
  wires:
  Block635/1 -> SubSystem278/1(element), SubSystem277/1(element), SubSystem276/1(element)
  SubSystem278/1(Output) -> Block636/4
  SubSystem277/1(Output) -> Block636/3
  SubSystem276/1(Output) -> Block636/2
  Block636/1 -> Block637/1
  Block634/1 -> Block636/1

== system lib_all/SubSystem176/SubSystem178/SubSystem182/SubSystem236/SubSystem245/SubSystem279/SubSystem280 ==
  note: ***編集禁止***
  element : Inport
  Inside_DSR : DataStoreRead  DataStoreName=DSM3
  Selector : Selector  IndexOptions=Index vector (port)  Indices=[1 3]  OutputSizes=1
  Output : Outport
  wires:
  Selector/1 -> Output/1
  element/1 -> Selector/2
  Inside_DSR/1 -> Selector/1

== system lib_all/SubSystem176/SubSystem178/SubSystem182/SubSystem236/SubSystem245/SubSystem279/SubSystem281 ==
  Block645 : Inport
  Block646 : Inport  Port=2
  Block647 : MultiPortSwitch  zeroidx=off  RndMeth=Zero  SaturateOnIntegerOverflow=on  InputSameDT=on
  SubSystem282 : Subsystem  TreatAsAtomicUnit=on  mask(***ATC.DSRVEC***){bd_version=1.01.A, r0=DSM7, r1=-1, r2=, r3=, r4=, r5=, r6=, r7=, r8=, r9=, c0=, c1=, c2=, c3=, c4=, c5=, c6=, c7=, c8=, c9=}
      mask prompts: bd_version "Version"
      mask help: これはAutoCからのブロックです．編集禁止．
      settings: OpenFcn=warndlg('変更禁止!');
      mask Help: web(ui_blockhelpmng(gcb));
      mask Display: disp('ATC.DSRVEC')
  SubSystem283 : Subsystem  TreatAsAtomicUnit=on  mask(***ATC.DSRVEC***){bd_version=1.01.A, r0=DSM6, r1=-1, r2=, r3=, r4=, r5=, r6=, r7=, r8=, r9=, c0=, c1=, c2=, c3=, c4=, c5=, c6=, c7=, c8=, c9=}
      mask prompts: bd_version "Version"
      mask help: これはAutoCからのブロックです．編集禁止．
      settings: OpenFcn=warndlg('変更禁止!');
      mask Help: web(ui_blockhelpmng(gcb));
      mask Display: disp('ATC.DSRVEC')
  SubSystem284 : Subsystem  TreatAsAtomicUnit=on  mask(***ATC.DSRVEC***){bd_version=1.01.A, r0=DSM19, r1=-1, r2=, r3=, r4=, r5=, r6=, r7=, r8=, r9=, c0=, c1=, c2=, c3=, c4=, c5=, c6=, c7=, c8=, c9=}
      mask prompts: bd_version "Version"
      mask help: これはAutoCからのブロックです．編集禁止．
      settings: OpenFcn=warndlg('変更禁止!');
      mask Help: web(ui_blockhelpmng(gcb));
      mask Display: disp('ATC.DSRVEC')
  Block648 : Outport
  wires:
  Block646/1 -> SubSystem284/1(element), SubSystem283/1(element), SubSystem282/1(element)
  SubSystem284/1(Output) -> Block647/4
  SubSystem283/1(Output) -> Block647/3
  SubSystem282/1(Output) -> Block647/2
  Block647/1 -> Block648/1
  Block645/1 -> Block647/1

== system lib_all/SubSystem176/SubSystem178/SubSystem182/SubSystem236/SubSystem245/SubSystem279/SubSystem285 ==
  Block649 : Inport
  Block650 : Inport  Port=2
  Block651 : MultiPortSwitch  zeroidx=off  RndMeth=Zero  SaturateOnIntegerOverflow=on  InputSameDT=on
  SubSystem286 : Subsystem  TreatAsAtomicUnit=on  mask(***ATC.DSRVEC***){bd_version=1.01.A, r0=DSM7, r1=-1, r2=, r3=, r4=, r5=, r6=, r7=, r8=, r9=, c0=, c1=, c2=, c3=, c4=, c5=, c6=, c7=, c8=, c9=}
      mask prompts: bd_version "Version"
      mask help: これはAutoCからのブロックです．編集禁止．
      settings: OpenFcn=warndlg('変更禁止!');
      mask Help: web(ui_blockhelpmng(gcb));
      mask Display: disp('ATC.DSRVEC')
  SubSystem287 : Subsystem  TreatAsAtomicUnit=on  mask(***ATC.DSRVEC***){bd_version=1.01.A, r0=DSM6, r1=-1, r2=, r3=, r4=, r5=, r6=, r7=, r8=, r9=, c0=, c1=, c2=, c3=, c4=, c5=, c6=, c7=, c8=, c9=}
      mask prompts: bd_version "Version"
      mask help: これはAutoCからのブロックです．編集禁止．
      settings: OpenFcn=warndlg('変更禁止!');
      mask Help: web(ui_blockhelpmng(gcb));
      mask Display: disp('ATC.DSRVEC')
  SubSystem288 : Subsystem  TreatAsAtomicUnit=on  mask(***ATC.DSRVEC***){bd_version=1.01.A, r0=DSM19, r1=-1, r2=, r3=, r4=, r5=, r6=, r7=, r8=, r9=, c0=, c1=, c2=, c3=, c4=, c5=, c6=, c7=, c8=, c9=}
      mask prompts: bd_version "Version"
      mask help: これはAutoCからのブロックです．編集禁止．
      settings: OpenFcn=warndlg('変更禁止!');
      mask Help: web(ui_blockhelpmng(gcb));
      mask Display: disp('ATC.DSRVEC')
  Block652 : Outport
  wires:
  Block650/1 -> SubSystem288/1(element), SubSystem287/1(element), SubSystem286/1(element)
  SubSystem288/1(Output) -> Block651/4
  SubSystem287/1(Output) -> Block651/3
  SubSystem286/1(Output) -> Block651/2
  Block651/1 -> Block652/1
  Block649/1 -> Block651/1

== system lib_all/SubSystem176/SubSystem303/SubSystem305/SubSystem308/SubSystem313/SubSystem314/SubSystem315 ==
  note: ***編集禁止***
  input : Inport
  element : Inport  Port=2
  Assignment : Assignment  IndexOptions=Index vector (port)  Indices=1  OutputSizes=[1 1]
  Inside_DSR : DataStoreRead  DataStoreName=DSM378
  Inside_DSW : DataStoreWrite  DataStoreName=DSM378
  wires:
  element/1 -> Assignment/3
  input/1 -> Assignment/2
  Inside_DSR/1 -> Assignment/1
  Assignment/1 -> Inside_DSW/1

== system lib_all/SubSystem1770/SubSystem1774/SubSystem1778/SubSystem1779/SubSystem1783/SubSystem1786/SubSystem1787 ==
  note: ***編集禁止***
  element : Inport
  Inside_DSR : DataStoreRead  DataStoreName=DSM7
  Selector : Selector  IndexOptions=Index vector (port)  Indices=[1 3]  OutputSizes=1
  Output : Outport
  wires:
  Selector/1 -> Output/1
  element/1 -> Selector/2
  Inside_DSR/1 -> Selector/1

== system lib_all/SubSystem1770/SubSystem1774/SubSystem1778/SubSystem1779/SubSystem1783/SubSystem1786/SubSystem1788 ==
  note: ***編集禁止***
  element : Inport
  Inside_DSR : DataStoreRead  DataStoreName=DSM6
  Selector : Selector  IndexOptions=Index vector (port)  Indices=[1 3]  OutputSizes=1
  Output : Outport
  wires:
  Inside_DSR/1 -> Selector/1
  element/1 -> Selector/2
  Selector/1 -> Output/1

== system lib_all/SubSystem1770/SubSystem1774/SubSystem1778/SubSystem1779/SubSystem1783/SubSystem1786/SubSystem1789 ==
  note: ***編集禁止***
  element : Inport
  Inside_DSR : DataStoreRead  DataStoreName=DSM19
  Selector : Selector  IndexOptions=Index vector (port)  Indices=[1 3]  OutputSizes=1
  Output : Outport
  wires:
  Selector/1 -> Output/1
  element/1 -> Selector/2
  Inside_DSR/1 -> Selector/1

== system lib_all/SubSystem1770/SubSystem1774/SubSystem1791/SubSystem1792/SubSystem1796/SubSystem1799/SubSystem1800 ==
  note: ***編集禁止***
  element : Inport
  Inside_DSR : DataStoreRead  DataStoreName=DSM7
  Selector : Selector  IndexOptions=Index vector (port)  Indices=[1 3]  OutputSizes=1
  Output : Outport
  wires:
  Selector/1 -> Output/1
  element/1 -> Selector/2
  Inside_DSR/1 -> Selector/1

== system lib_all/SubSystem1770/SubSystem1774/SubSystem1791/SubSystem1792/SubSystem1796/SubSystem1799/SubSystem1801 ==
  note: ***編集禁止***
  element : Inport
  Inside_DSR : DataStoreRead  DataStoreName=DSM6
  Selector : Selector  IndexOptions=Index vector (port)  Indices=[1 3]  OutputSizes=1
  Output : Outport
  wires:
  Inside_DSR/1 -> Selector/1
  element/1 -> Selector/2
  Selector/1 -> Output/1

== system lib_all/SubSystem1770/SubSystem1774/SubSystem1791/SubSystem1792/SubSystem1796/SubSystem1799/SubSystem1802 ==
  note: ***編集禁止***
  element : Inport
  Inside_DSR : DataStoreRead  DataStoreName=DSM19
  Selector : Selector  IndexOptions=Index vector (port)  Indices=[1 3]  OutputSizes=1
  Output : Outport
  wires:
  Selector/1 -> Output/1
  element/1 -> Selector/2
  Inside_DSR/1 -> Selector/1

== system lib_all/SubSystem1770/SubSystem1774/SubSystem1804/SubSystem1805/SubSystem1809/SubSystem1812/SubSystem1813 ==
  note: ***編集禁止***
  element : Inport
  Inside_DSR : DataStoreRead  DataStoreName=DSM7
  Selector : Selector  IndexOptions=Index vector (port)  Indices=[1 3]  OutputSizes=1
  Output : Outport
  wires:
  Selector/1 -> Output/1
  element/1 -> Selector/2
  Inside_DSR/1 -> Selector/1

== system lib_all/SubSystem1770/SubSystem1774/SubSystem1804/SubSystem1805/SubSystem1809/SubSystem1812/SubSystem1814 ==
  note: ***編集禁止***
  element : Inport
  Inside_DSR : DataStoreRead  DataStoreName=DSM6
  Selector : Selector  IndexOptions=Index vector (port)  Indices=[1 3]  OutputSizes=1
  Output : Outport
  wires:
  Inside_DSR/1 -> Selector/1
  element/1 -> Selector/2
  Selector/1 -> Output/1

== system lib_all/SubSystem1770/SubSystem1774/SubSystem1804/SubSystem1805/SubSystem1809/SubSystem1812/SubSystem1815 ==
  note: ***編集禁止***
  element : Inport
  Inside_DSR : DataStoreRead  DataStoreName=DSM19
  Selector : Selector  IndexOptions=Index vector (port)  Indices=[1 3]  OutputSizes=1
  Output : Outport
  wires:
  Selector/1 -> Output/1
  element/1 -> Selector/2
  Inside_DSR/1 -> Selector/1

== system lib_all/SubSystem1770/SubSystem1817/SubSystem1821/SubSystem1822/SubSystem1826/SubSystem1829/SubSystem1830 ==
  note: ***編集禁止***
  element : Inport
  Inside_DSR : DataStoreRead  DataStoreName=DSM7
  Selector : Selector  IndexOptions=Index vector (port)  Indices=[1 3]  OutputSizes=1
  Output : Outport
  wires:
  Selector/1 -> Output/1
  element/1 -> Selector/2
  Inside_DSR/1 -> Selector/1

== system lib_all/SubSystem1770/SubSystem1817/SubSystem1821/SubSystem1822/SubSystem1826/SubSystem1829/SubSystem1831 ==
  note: ***編集禁止***
  element : Inport
  Inside_DSR : DataStoreRead  DataStoreName=DSM6
  Selector : Selector  IndexOptions=Index vector (port)  Indices=[1 3]  OutputSizes=1
  Output : Outport
  wires:
  Inside_DSR/1 -> Selector/1
  element/1 -> Selector/2
  Selector/1 -> Output/1
